simulink model "CCMHfaMotor"  (R2022b)
settings: Solver=FixedStepDiscrete  StartTime=0.0  StopTime=10.0  FixedStep=0.01  RelTol=1e-3
model workspace (MAT data in the model file):
  HfaWakeUpFlag = Simulink.Signal
  HfaWakeUpFlag.DataType = 'UInt8'
  HfaWakeUpFlag.Dimensions = 1
  HfaWakeUpFlag.DimensionsMode = 'auto'
  HfaWakeUpFlag.Complexity = 'real'
  HfaWakeUpFlag.SampleTime = -1
  HfaWakeUpFlag.SamplingMode = 'auto'
  NvMBlockHfaMtrCalData = Simulink.Signal
  NvMBlockHfaMtrCalData.DataType = 'UInt8'
  NvMBlockHfaMtrCalData.Dimensions = [200 1]
  NvMBlockHfaMtrCalData.DimensionsMode = 'auto'
  NvMBlockHfaMtrCalData.Complexity = 'real'
  NvMBlockHfaMtrCalData.SampleTime = -1
  NvMBlockHfaMtrCalData.SamplingMode = 'auto'
  NvMBlockHfaMtrCalData.InitialValue = 'NvMBlockHfaMtrCalDataInit'
  NvMBlockHfaMtrCalDataInit = Simulink.Parameter
  NvMBlockHfaMtrCalDataInit.Value = [0; 0; 0; 0; 0; 0; 0; 0; 0; 0; 0; 0; 0; 0; 0; 0; 0; 0; 0; 0; 0; 0; 0; 0; 0; 0; 0; 0; 0; 0; 0; 0; 0; 0; 0; 0; 0; 0; 0; 0; 0; 0; 0; 0; 0; 0; 0; 0; 0; 0; 0; 0; 0; 0; 0; 0; 0; 0; 0; 0; 0; 0; 0; 0; 0; 0; 0; 0; 0; 0; 0; 0; 0; 0; 0; 0; 0; 0; 0; 0; 0; 0; 0; 0; 0; 0; 0; 0; 0; 0; 0; 0; 0; 0; 0; 0; 0; 0; 0; 0; 0; 0; 0; 0; 0; 0; 0; 0; 0; 0; 0; 0; 0; 0; 0; 0; 0; 0; 0; 0; 0; 0; 0; 0; 0; 0; 0; 0; 0; 0; 0; 0; 0; 0; 0; 0; 0; 0; 0; 0; 0; 0; 0; 0; 0; 0; 0; 0; 0; 0; 0; 0; 0; 0; 0; 0; 0; 0; 0; 0; 0; 0; 0; 0; 0; 0; 0; 0; 0; 0; 0; 0; 0; 0; 0; 0; 0; 0; 0; 0; 0; 0; 0; 0; 0; 0; 0; 0; 0; 0; 0; 0; 0; 0; 0; 0; 0; 0; 0; 0]
  NvMBlockHfaMtrCalDataInit.DataType = 'UInt8'
  NvMBlockHfaMtrCalDataInit.Complexity = 'real'
  NvMBlockHfaMtrCalDataInit.Dimensions = [200 1]
undefined symbols (not defined in the file or in its folder): ComChlType, DioChannelType, HFAChlType, LinFrameType, NetworkChlType, NvMBlockId, P_DioLevelType, P_LinIfSchHandleType, P_Std_ReturnType, PowerChlType
(part 1 of 4)
settings Model: Creator=blue.ge  LastModifiedBy=blue.ge
settings DataIOCC: SaveFormat=StructureWithTime
settings OptimizationCC: DefaultParameterBehavior=Inlined  EnhancedBackFolding=on  PassReuseOutputArgsAs=Individual arguments  ZeroExternalMemoryAtStartup=off  ZeroInternalMemoryAtStartup=off  OptimizeBlockOrder=speed  DifferentSizesBufferReuse=on  OptimizationCustomize=off  EfficientTunableParamExpr=on
settings DebuggingCC: ParameterTunabilityLossMsg=error
settings ModelReferenceCC: ModelReferenceNumInstancesAllowed=Single
settings SFSimCC: MATLABDynamicMemAlloc=off
settings RTWCC: Description=AUTOSAR  SystemTargetFile=autosar.tlc  GenCodeOnly=on  TemplateMakefile=ert_default_tmf
settings CodeAppCC: ForceParamTrailComments=on  IgnoreCustomStorageClasses=off  OperatorAnnotations=on  SimulinkDataObjDesc=on  SFDataObjDesc=on  InsertBlockDesc=on
settings STFCustomTargetCC: TargetLangStandard=C99 (ISO)  MultiwordLength=256  ParMdlRefBuildCompliant=on  CompOptLevelCompliant=on  IncludeMdlTerminateFcn=off  CombineOutputUpdateFcns=on  SuppressErrorStatus=on  ERTCustomFileBanners=on  SupportNonFinite=off  SupportComplex=off  SupportContinuousTime=off  SupportNonInlinedSFcns=off  RemoveResetFunc=on  MATLABClassNameForMDSCustomization=Simulink.SoftwareTarget.ERTCustomization  AutosarCompliant=on  UseToolchainInfoCompliant=on  LookupTableObjectStructAxisOrder=2,1,3,4,...  LUTObjectStructOrderEvenSpacing=Size,Table,Breakpoints  SystemTargetFile=autosar.tlc
settings ERTTargetCC: TargetLangStandard=C99 (ISO)  ConcurrentExecutionCompliant=off  IncludeMdlTerminateFcn=off  SuppressErrorStatus=on  SupportNonFinite=off  SupportComplex=off  ModelStepFunctionPrototypeControlCompliant=off  CPPClassGenCompliant=off  AutosarCompliant=on  LookupTableObjectStructAxisOrder=2,1,3,4,...  LUTObjectStructOrderEvenSpacing=Size,Table,Breakpoints  InlinedParameterPlacement=NonHierarchical  ERTCustomFileTemplate=example_file_process.tlc

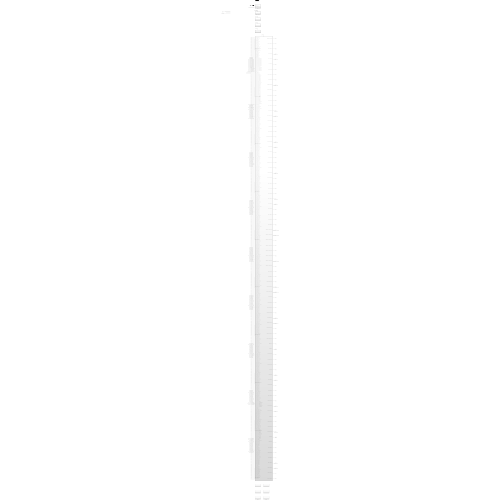
[thumbnail: Simulink's stored preview of the top-level diagram]
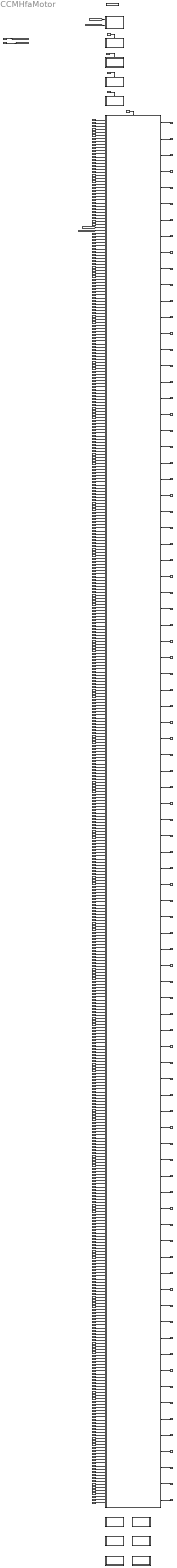
== system CCMHfaMotor ==
  CCMHfaMotor_HfaMotMode : Inport  OutputFunctionCall=on
  CCMHfaMotor_ReHvacMotMode : Inport  Port=2  OutputFunctionCall=on
  CCMHfaMotor_WtrMotMode : Inport  Port=3  OutputFunctionCall=on
  HfaMotor_50msRunnable : Inport  Port=4  SampleTime=0.05  OutputFunctionCall=on
  HfaMotor_Runnable : Inport  Port=5  SampleTime=0.02  OutputFunctionCall=on
  SbcPwrOn_SbcPwrOn : Inport  Port=6  OutDataTypeStr=PwrOnff  PortDimensions=1
  SbcWakeupSta_SbcWakeupSta : Inport  Port=7  OutDataTypeStr=Enum: SbcWakeupSta  PortDimensions=1
  AcRfrPrgSafety_AcRfrPrgSafety : Inport  Port=8  OutDataTypeStr=Enum: ActCfrPrgSafty  PortDimensions=1
  ActRfrIdeGroup_ActRfrIdeGroup : Inport  Port=9  OutDataTypeStr=Enum: ActCfrGroup  PortDimensions=1
  ActRfrIdeItem_ActRfrIdeItem : Inport  Port=10  OutDataTypeStr=Uint5  PortDimensions=1
  ActRfrIdePN_ActRfrIdePN : Inport  Port=11  OutDataTypeStr=UInt8  PortDimensions=1
  ActRfrIdeSupplier_ActRfrIdeSupplier : Inport  Port=12  OutDataTypeStr=UInt8  PortDimensions=1
  ActRfrIdeVers_ActRfrIdeVers : Inport  Port=13  OutDataTypeStr=UInt8  PortDimensions=1
  ActRfrPrgDiret_ActRfrPrgDiret : Inport  Port=14  OutDataTypeStr=Enum: ActCfrPrgDir  PortDimensions=1
  ActRfrPrgGroup_ActRfrPrgGroup : Inport  Port=15  OutDataTypeStr=Enum: ActCfrGroup  PortDimensions=1
  ActRfrPrgItem_ActRfrPrgItem : Inport  Port=16  OutDataTypeStr=Uint5  PortDimensions=1
  ActRfrPrgPrgGroup_ActRfrPrgPrgGroup : Inport  Port=17  OutDataTypeStr=Enum: ActCfrGroup  PortDimensions=1
  ActRfrPrgPrgItem_ActRfrPrgPrgItem : Inport  Port=18  OutDataTypeStr=Uint5  PortDimensions=1
  ActRfrPrgRef_ActRfrPrgRef : Inport  Port=19  OutDataTypeStr=UInt8  PortDimensions=1
  ActRfrPrgSafetyEnable_ActRfrPrgSafetyEnable : Inport  Port=20  OutDataTypeStr=Enum: ActvInActv  PortDimensions=1
  ActRfrStaCPos_ActRfrStaCPos : Inport  Port=21  OutDataTypeStr=UInt15  PortDimensions=1
  ActRfrStaEmgyRun_ActRfrStaEmgyRun : Inport  Port=22  OutDataTypeStr=Enum: ActvInActv  PortDimensions=1
  ActRfrStaErr_ActRfrStaErr : Inport  Port=23  OutDataTypeStr=Enum: ActRfrErr  PortDimensions=1
  ActRfrStaGroup_ActRfrStaGroup : Inport  Port=24  OutDataTypeStr=Enum: ActCfrGroup  PortDimensions=1
  ActRfrStaItem_ActRfrStaItem : Inport  Port=25  OutDataTypeStr=Uint5  PortDimensions=1
  ActRfrStaMod_ActRfrStaMod : Inport  Port=26  OutDataTypeStr=Enum: ActRfrMod  PortDimensions=1
  ActRfrStaReset_ActRfrStaReset : Inport  Port=27  OutDataTypeStr=Enum: ReqSts1  PortDimensions=1
  ActRfrStaStd_ActRfrStaStd : Inport  Port=28  OutDataTypeStr=Enum: YesNo2  PortDimensions=1
  BatSysBtrMSts_BatSysBtrMSts : Inport  Port=29  OutDataTypeStr=Enum: BatBtrMStsEnum  PortDimensions=1
  HfaCcmSlaveRespB0Lin4_HfaCcmSlaveRespB0Lin4 : Inport  Port=30  OutDataTypeStr=UInt8  PortDimensions=1
  HfaCcmSlaveRespB1Lin4_HfaCcmSlaveRespB1Lin4 : Inport  Port=31  OutDataTypeStr=UInt8  PortDimensions=1
  HfaCcmSlaveRespB2Lin4_HfaCcmSlaveRespB2Lin4 : Inport  Port=32  OutDataTypeStr=UInt8  PortDimensions=1
  HfaCcmSlaveRespB3Lin4_HfaCcmSlaveRespB3Lin4 : Inport  Port=33  OutDataTypeStr=UInt8  PortDimensions=1
  HfaCcmSlaveRespB4Lin4_HfaCcmSlaveRespB4Lin4 : Inport  Port=34  OutDataTypeStr=UInt8  PortDimensions=1
  HfaCcmSlaveRespB5Lin4_HfaCcmSlaveRespB5Lin4 : Inport  Port=35  OutDataTypeStr=UInt8  PortDimensions=1
  HfaCcmSlaveRespB6Lin4_HfaCcmSlaveRespB6Lin4 : Inport  Port=36  OutDataTypeStr=UInt8  PortDimensions=1
  HfaCcmSlaveRespB7Lin4_HfaCcmSlaveRespB7Lin4 : Inport  Port=37  OutDataTypeStr=UInt8  PortDimensions=1
  HmiElecAirSwngReq_HmiElecAirSwngReq : Inport  Port=38  OutDataTypeStr=Bus: HmiElecAirSwngReq  PortDimensions=1  BusOutputAsStruct=on
  HvacCfgForClimaCtrl_HvacCfgForHvacFrontBlowerType : Inport  Port=39  OutDataTypeStr=HvacCfgForHvacFrontBlowerType  PortDimensions=1
  HvacCfgForClimaCtrl_HvacCfgForHvacMiscExistSts : Inport  Port=40  OutDataTypeStr=HvacCfgForHvacMiscExistSts  PortDimensions=1
  HvacCfgForClimaCtrl_HvacCfgForHvacMotorExistSts : Inport  Port=41  OutDataTypeStr=HvacCfgForHvacMotorExistSts  PortDimensions=1
  HvacCfgForClimaCtrl_HvacCfgForHvacSensorExistSts : Inport  Port=42  OutDataTypeStr=HvacCfgForHvacSensorExistSts  PortDimensions=1
  SysServCarCfgSts_SysServCarID : Inport  Port=43  OutDataTypeStr=Enum: SysServCarID  PortDimensions=1
  SysServCarCfgSts_SysServCCPAQSSts : Inport  Port=44  OutDataTypeStr=UInt8  PortDimensions=1
  SysServCarCfgSts_SysServCCPHEXSensorSts : Inport  Port=45  OutDataTypeStr=UInt8  PortDimensions=1
  SysServCarCfgSts_SysServCCPLin2AFUSts : Inport  Port=46  OutDataTypeStr=Boolean  PortDimensions=1
  SysServCarCfgSts_SysServCCPLin2AIUSts : Inport  Port=47  OutDataTypeStr=UInt8  PortDimensions=1
  SysServCarCfgSts_SysServCCPLin2CDSSts : Inport  Port=48  OutDataTypeStr=Boolean  PortDimensions=1
  SysServCarCfgSts_SysServCCPLin2PMSISts : Inport  Port=49  OutDataTypeStr=Boolean  PortDimensions=1
  SysServCarCfgSts_SysServCCPLin3VGASts : Inport  Port=50  OutDataTypeStr=Boolean  PortDimensions=1
  SysServCarCfgSts_SysServDUAirVentSnrSts : Inport  Port=51  OutDataTypeStr=Boolean  PortDimensions=1
  SysServCarCfgSts_SysServDUBlowerPwmSignSts : Inport  Port=52  OutDataTypeStr=Boolean  PortDimensions=1
  SysServCarCfgSts_SysServDUBlowerPwmSts : Inport  Port=53  OutDataTypeStr=Boolean  PortDimensions=1
  SysServCarCfgSts_SysServDUDCDefrostSts : Inport  Port=54  OutDataTypeStr=UInt8  PortDimensions=1
  SysServCarCfgSts_SysServDUDCModeSts : Inport  Port=55  OutDataTypeStr=UInt8  PortDimensions=1
  SysServCarCfgSts_SysServDUDCRecSts : Inport  Port=56  OutDataTypeStr=UInt8  PortDimensions=1
  SysServCarCfgSts_SysServDUDCSModeSts : Inport  Port=57  OutDataTypeStr=UInt8  PortDimensions=1
  SysServCarCfgSts_SysServDUDCSTLSts : Inport  Port=58  OutDataTypeStr=UInt8  PortDimensions=1
  SysServCarCfgSts_SysServDUDCTLSts : Inport  Port=59  OutDataTypeStr=UInt8  PortDimensions=1
  SysServCarCfgSts_SysServDUDCTRSts : Inport  Port=60  OutDataTypeStr=UInt8  PortDimensions=1
  SysServCarCfgSts_SysServDUFLFootSts : Inport  Port=61  OutDataTypeStr=UInt8  PortDimensions=1
  SysServCarCfgSts_SysServDUFLVentSts : Inport  Port=62  OutDataTypeStr=UInt8  PortDimensions=1
  SysServCarCfgSts_SysServDUFRFootSts : Inport  Port=63  OutDataTypeStr=UInt8  PortDimensions=1
  SysServCarCfgSts_SysServDUFRVentSts : Inport  Port=64  OutDataTypeStr=UInt8  PortDimensions=1
  SysServCarCfgSts_SysServDUIncarMotorSts : Inport  Port=65  OutDataTypeStr=Boolean  PortDimensions=1
  SysServCarCfgSts_SysServDULin1HBMFSts : Inport  Port=66  OutDataTypeStr=Boolean  PortDimensions=1
  SysServCarCfgSts_SysServDULin4HFAFSts : Inport  Port=67  OutDataTypeStr=UInt32  PortDimensions=1
  SysServCarCfgSts_SysServDUSFLFootSts : Inport  Port=68  OutDataTypeStr=UInt8  PortDimensions=1
  SysServCarCfgSts_SysServDUSFLVentSts : Inport  Port=69  OutDataTypeStr=UInt8  PortDimensions=1
  SysServCarCfgSts_SysServDUSFRFootSts : Inport  Port=70  OutDataTypeStr=UInt8  PortDimensions=1
  SysServCarCfgSts_SysServDUSFRVentSts : Inport  Port=71  OutDataTypeStr=UInt8  PortDimensions=1
  SysServCarCfgSts_SysServDUSnsrForFCmptmtTSts : Inport  Port=72  OutDataTypeStr=UInt8  PortDimensions=1
  SysServCarCfgSts_SysServDUSnsrForRair : Inport  Port=73  OutDataTypeStr=UInt8  PortDimensions=1
  SysServCarCfgSts_SysServDUSnsrForRCmptmtTSts : Inport  Port=74  OutDataTypeStr=UInt8  PortDimensions=1
  BatLoadBtrMSts_BatLoadBtrMSts : Inport  Port=75  OutDataTypeStr=Enum: BatLoadBtrMStsEnum  PortDimensions=1
  HfaMotSetPowerOnInit_PowerOnInit : Inport  Port=76  OutDataTypeStr=Boolean  PortDimensions=1
  VehModMngtGlbSafe1_VehModMngtGlbSafe1 : Inport  Port=77  OutDataTypeStr=Bus: VehModMngtGlbSafe1  PortDimensions=1  BusOutputAsStruct=on
  HfaActr01ActPson_HfaActr01ActPson : Inport  Port=78  OutDataTypeStr=UInt16  PortDimensions=1
  HfaActr01ActPson_HfaActr01ActPson_IsUpdated : Inport  Port=79  OutDataTypeStr=boolean  PortDimensions=1
  HfaActr01BlckInd_HfaActr01BlckInd : Inport  Port=80  OutDataTypeStr=Enum: VentnActr01BlckInd  PortDimensions=1
  HfaActr01BlckSts_HfaActr01BlckSts : Inport  Port=81  OutDataTypeStr=Enum: VentnActr01BlckSts  PortDimensions=1
  HfaActr01BusAdr_HfaActr01BusAdr : Inport  Port=82  OutDataTypeStr=UInt8  PortDimensions=1
  HfaActr01CoilSts_HfaActr01CoilSts : Inport  Port=83  OutDataTypeStr=Enum: VentnMotUrgcUnlck  PortDimensions=1
  HfaActr01DrvDir_HfaActr01DrvDir : Inport  Port=84  OutDataTypeStr=Enum: VentnActr01DrvDir  PortDimensions=1
  HfaActr01ElecErr_HfaActr01ElecErr : Inport  Port=85  OutDataTypeStr=Enum: VentnActr01ElecErr  PortDimensions=1
  HfaActr01Mode_HfaActr01Mode : Inport  Port=86  OutDataTypeStr=Enum: VentnMotMode  PortDimensions=1
  HfaActr01MovDir_HfaActr01MovDir : Inport  Port=87  OutDataTypeStr=Enum: VentnMotDir  PortDimensions=1
  HfaActr01OvrTempErr_HfaActr01OvrTempErr : Inport  Port=88  OutDataTypeStr=Enum: VentnActr01OvrTempErr  PortDimensions=1
  HfaActr01PsonSts_HfaActr01PsonSts : Inport  Port=89  OutDataTypeStr=Enum: VentnMotPsonAvl  PortDimensions=1
  HfaActr01ResetSts_HfaActr01ResetSts : Inport  Port=90  OutDataTypeStr=Enum: VentnActr01ResetSts  PortDimensions=1
  HfaActr01SpclFct_HfaActr01SpclFct : Inport  Port=91  OutDataTypeStr=Enum: VentnActr01SpclFct  PortDimensions=1
  HfaActr01SpdSts_HfaActr01SpdSts : Inport  Port=92  OutDataTypeStr=Enum: VentnActr01SpdSts  PortDimensions=1
  HfaActr01TrqSts_HfaActr01TrqSts : Inport  Port=93  OutDataTypeStr=Enum: VentnMotUrgcUnlck  PortDimensions=1
  HfaActr01UrgcExist_HfaActr01UrgcExist : Inport  Port=94  OutDataTypeStr=Enum: VentnActr01UrgcExist  PortDimensions=1
  HfaActr01UrgcPson_HfaActr01UrgcPson : Inport  Port=95  OutDataTypeStr=Enum: VentnMotUrgcPson  PortDimensions=1
  HfaActr01UrgcSts_HfaActr01UrgcSts : Inport  Port=96  OutDataTypeStr=Enum: VentnMotUrgcUnlck  PortDimensions=1
  HfaActr01VoltErr_HfaActr01VoltErr : Inport  Port=97  OutDataTypeStr=Enum: VentnActr01VoltErr  PortDimensions=1
  HfaActr02ActPson_HfaActr02ActPson : Inport  Port=98  OutDataTypeStr=UInt16  PortDimensions=1
  HfaActr02ActPson_HfaActr02ActPson_IsUpdated : Inport  Port=99  OutDataTypeStr=boolean  PortDimensions=1
  HfaActr02BlckInd_HfaActr02BlckInd : Inport  Port=100  OutDataTypeStr=Enum: VentnActr01BlckInd  PortDimensions=1
  HfaActr02BlckSts_HfaActr02BlckSts : Inport  Port=101  OutDataTypeStr=Enum: VentnActr01BlckSts  PortDimensions=1
  HfaActr02BusAdr_HfaActr02BusAdr : Inport  Port=102  OutDataTypeStr=UInt8  PortDimensions=1
  HfaActr02CoilSts_HfaActr02CoilSts : Inport  Port=103  OutDataTypeStr=Enum: VentnMotUrgcUnlck  PortDimensions=1
  HfaActr02DrvDir_HfaActr02DrvDir : Inport  Port=104  OutDataTypeStr=Enum: VentnActr01DrvDir  PortDimensions=1
  HfaActr02ElecErr_HfaActr02ElecErr : Inport  Port=105  OutDataTypeStr=Enum: VentnActr01ElecErr  PortDimensions=1
  HfaActr02Mode_HfaActr02Mode : Inport  Port=106  OutDataTypeStr=Enum: VentnMotMode  PortDimensions=1
  HfaActr02MovDir_HfaActr02MovDir : Inport  Port=107  OutDataTypeStr=Enum: VentnMotDir  PortDimensions=1
  HfaActr02OvrTempErr_HfaActr02OvrTempErr : Inport  Port=108  OutDataTypeStr=Enum: VentnActr01OvrTempErr  PortDimensions=1
  HfaActr02PsonSts_HfaActr02PsonSts : Inport  Port=109  OutDataTypeStr=Enum: VentnMotPsonAvl  PortDimensions=1
  HfaActr02ResetSts_HfaActr02ResetSts : Inport  Port=110  OutDataTypeStr=Enum: VentnActr01ResetSts  PortDimensions=1
  HfaActr02SpclFct_HfaActr02SpclFct : Inport  Port=111  OutDataTypeStr=Enum: VentnActr01SpclFct  PortDimensions=1
  HfaActr02SpdSts_HfaActr02SpdSts : Inport  Port=112  OutDataTypeStr=Enum: VentnActr01SpdSts  PortDimensions=1
  HfaActr02TrqSts_HfaActr02TrqSts : Inport  Port=113  OutDataTypeStr=Enum: VentnMotUrgcUnlck  PortDimensions=1
  HfaActr02UrgcExist_HfaActr02UrgcExist : Inport  Port=114  OutDataTypeStr=Enum: VentnActr01UrgcExist  PortDimensions=1
  HfaActr02UrgcPson_HfaActr02UrgcPson : Inport  Port=115  OutDataTypeStr=Enum: VentnMotUrgcPson  PortDimensions=1
  HfaActr02UrgcSts_HfaActr02UrgcSts : Inport  Port=116  OutDataTypeStr=Enum: VentnMotUrgcUnlck  PortDimensions=1
  HfaActr02VoltErr_HfaActr02VoltErr : Inport  Port=117  OutDataTypeStr=Enum: VentnActr01VoltErr  PortDimensions=1
  HfaActr03ActPson_HfaActr03ActPson : Inport  Port=118  OutDataTypeStr=UInt16  PortDimensions=1
  HfaActr03ActPson_HfaActr03ActPson_IsUpdated : Inport  Port=119  OutDataTypeStr=boolean  PortDimensions=1
  HfaActr03BlckInd_HfaActr03BlckInd : Inport  Port=120  OutDataTypeStr=Enum: VentnActr01BlckInd  PortDimensions=1
  HfaActr03BlckSts_HfaActr03BlckSts : Inport  Port=121  OutDataTypeStr=Enum: VentnActr01BlckSts  PortDimensions=1
  HfaActr03BusAdr_HfaActr03BusAdr : Inport  Port=122  OutDataTypeStr=UInt8  PortDimensions=1
  HfaActr03CoilSts_HfaActr03CoilSts : Inport  Port=123  OutDataTypeStr=Enum: VentnMotUrgcUnlck  PortDimensions=1
  HfaActr03DrvDir_HfaActr03DrvDir : Inport  Port=124  OutDataTypeStr=Enum: VentnActr01DrvDir  PortDimensions=1
  HfaActr03ElecErr_HfaActr03ElecErr : Inport  Port=125  OutDataTypeStr=Enum: VentnActr01ElecErr  PortDimensions=1
  HfaActr03Mode_HfaActr03Mode : Inport  Port=126  OutDataTypeStr=Enum: VentnMotMode  PortDimensions=1
  HfaActr03MovDir_HfaActr03MovDir : Inport  Port=127  OutDataTypeStr=Enum: VentnMotDir  PortDimensions=1
  HfaActr03OvrTempErr_HfaActr03OvrTempErr : Inport  Port=128  OutDataTypeStr=Enum: VentnActr01OvrTempErr  PortDimensions=1
  HfaActr03PsonSts_HfaActr03PsonSts : Inport  Port=129  OutDataTypeStr=Enum: VentnMotPsonAvl  PortDimensions=1
  HfaActr03ResetSts_HfaActr03ResetSts : Inport  Port=130  OutDataTypeStr=Enum: VentnActr01ResetSts  PortDimensions=1
  HfaActr03SpclFct_HfaActr03SpclFct : Inport  Port=131  OutDataTypeStr=Enum: VentnActr01SpclFct  PortDimensions=1
  HfaActr03SpdSts_HfaActr03SpdSts : Inport  Port=132  OutDataTypeStr=Enum: VentnActr01SpdSts  PortDimensions=1
  HfaActr03TrqSts_HfaActr03TrqSts : Inport  Port=133  OutDataTypeStr=Enum: VentnMotUrgcUnlck  PortDimensions=1
  HfaActr03UrgcExist_HfaActr03UrgcExist : Inport  Port=134  OutDataTypeStr=Enum: VentnActr01UrgcExist  PortDimensions=1
  HfaActr03UrgcPson_HfaActr03UrgcPson : Inport  Port=135  OutDataTypeStr=Enum: VentnMotUrgcPson  PortDimensions=1
  HfaActr03UrgcSts_HfaActr03UrgcSts : Inport  Port=136  OutDataTypeStr=Enum: VentnMotUrgcUnlck  PortDimensions=1
  HfaActr03VoltErr_HfaActr03VoltErr : Inport  Port=137  OutDataTypeStr=Enum: VentnActr01VoltErr  PortDimensions=1
  HfaActr04ActPson_HfaActr04ActPson : Inport  Port=138  OutDataTypeStr=UInt16  PortDimensions=1
  HfaActr04ActPson_HfaActr04ActPson_IsUpdated : Inport  Port=139  OutDataTypeStr=boolean  PortDimensions=1
  HfaActr04BlckInd_HfaActr04BlckInd : Inport  Port=140  OutDataTypeStr=Enum: VentnActr01BlckInd  PortDimensions=1
  HfaActr04BlckSts_HfaActr04BlckSts : Inport  Port=141  OutDataTypeStr=Enum: VentnActr01BlckSts  PortDimensions=1
  HfaActr04BusAdr_HfaActr04BusAdr : Inport  Port=142  OutDataTypeStr=UInt8  PortDimensions=1
  HfaActr04CoilSts_HfaActr04CoilSts : Inport  Port=143  OutDataTypeStr=Enum: VentnMotUrgcUnlck  PortDimensions=1
  HfaActr04DrvDir_HfaActr04DrvDir : Inport  Port=144  OutDataTypeStr=Enum: VentnActr01DrvDir  PortDimensions=1
  HfaActr04ElecErr_HfaActr04ElecErr : Inport  Port=145  OutDataTypeStr=Enum: VentnActr01ElecErr  PortDimensions=1
  HfaActr04Mode_HfaActr04Mode : Inport  Port=146  OutDataTypeStr=Enum: VentnMotMode  PortDimensions=1
  HfaActr04MovDir_HfaActr04MovDir : Inport  Port=147  OutDataTypeStr=Enum: VentnMotDir  PortDimensions=1
  HfaActr04OvrTempErr_HfaActr04OvrTempErr : Inport  Port=148  OutDataTypeStr=Enum: VentnActr01OvrTempErr  PortDimensions=1
  HfaActr04PsonSts_HfaActr04PsonSts : Inport  Port=149  OutDataTypeStr=Enum: VentnMotPsonAvl  PortDimensions=1
  HfaActr04ResetSts_HfaActr04ResetSts : Inport  Port=150  OutDataTypeStr=Enum: VentnActr01ResetSts  PortDimensions=1
  HfaActr04SpclFct_HfaActr04SpclFct : Inport  Port=151  OutDataTypeStr=Enum: VentnActr01SpclFct  PortDimensions=1
  HfaActr04SpdSts_HfaActr04SpdSts : Inport  Port=152  OutDataTypeStr=Enum: VentnActr01SpdSts  PortDimensions=1
  HfaActr04TrqSts_HfaActr04TrqSts : Inport  Port=153  OutDataTypeStr=Enum: VentnMotUrgcUnlck  PortDimensions=1
  HfaActr04UrgcExist_HfaActr04UrgcExist : Inport  Port=154  OutDataTypeStr=Enum: VentnActr01UrgcExist  PortDimensions=1
  HfaActr04UrgcPson_HfaActr04UrgcPson : Inport  Port=155  OutDataTypeStr=Enum: VentnMotUrgcPson  PortDimensions=1
  HfaActr04UrgcSts_HfaActr04UrgcSts : Inport  Port=156  OutDataTypeStr=Enum: VentnMotUrgcUnlck  PortDimensions=1
  HfaActr04VoltErr_HfaActr04VoltErr : Inport  Port=157  OutDataTypeStr=Enum: VentnActr01VoltErr  PortDimensions=1
  HfaActr05ActPson_HfaActr05ActPson : Inport  Port=158  OutDataTypeStr=UInt16  PortDimensions=1
  HfaActr05ActPson_HfaActr05ActPson_IsUpdated : Inport  Port=159  OutDataTypeStr=boolean  PortDimensions=1
  HfaActr05BlckInd_HfaActr05BlckInd : Inport  Port=160  OutDataTypeStr=Enum: VentnActr01BlckInd  PortDimensions=1
  HfaActr05BlckSts_HfaActr05BlckSts : Inport  Port=161  OutDataTypeStr=Enum: VentnActr01BlckSts  PortDimensions=1
  HfaActr05BusAdr_HfaActr05BusAdr : Inport  Port=162  OutDataTypeStr=UInt8  PortDimensions=1
  HfaActr05CoilSts_HfaActr05CoilSts : Inport  Port=163  OutDataTypeStr=Enum: VentnMotUrgcUnlck  PortDimensions=1
  HfaActr05DrvDir_HfaActr05DrvDir : Inport  Port=164  OutDataTypeStr=Enum: VentnActr01DrvDir  PortDimensions=1
  HfaActr05ElecErr_HfaActr05ElecErr : Inport  Port=165  OutDataTypeStr=Enum: VentnActr01ElecErr  PortDimensions=1
  HfaActr05Mode_HfaActr05Mode : Inport  Port=166  OutDataTypeStr=Enum: VentnMotMode  PortDimensions=1
  HfaActr05MovDir_HfaActr05MovDir : Inport  Port=167  OutDataTypeStr=Enum: VentnMotDir  PortDimensions=1
  HfaActr05OvrTempErr_HfaActr05OvrTempErr : Inport  Port=168  OutDataTypeStr=Enum: VentnActr01OvrTempErr  PortDimensions=1
  HfaActr05PsonSts_HfaActr05PsonSts : Inport  Port=169  OutDataTypeStr=Enum: VentnMotPsonAvl  PortDimensions=1
  HfaActr05ResetSts_HfaActr05ResetSts : Inport  Port=170  OutDataTypeStr=Enum: VentnActr01ResetSts  PortDimensions=1
  HfaActr05SpclFct_HfaActr05SpclFct : Inport  Port=171  OutDataTypeStr=Enum: VentnActr01SpclFct  PortDimensions=1
  HfaActr05SpdSts_HfaActr05SpdSts : Inport  Port=172  OutDataTypeStr=Enum: VentnActr01SpdSts  PortDimensions=1
  HfaActr05TrqSts_HfaActr05TrqSts : Inport  Port=173  OutDataTypeStr=Enum: VentnMotUrgcUnlck  PortDimensions=1
  HfaActr05UrgcExist_HfaActr05UrgcExist : Inport  Port=174  OutDataTypeStr=Enum: VentnActr01UrgcExist  PortDimensions=1
  HfaActr05UrgcPson_HfaActr05UrgcPson : Inport  Port=175  OutDataTypeStr=Enum: VentnMotUrgcPson  PortDimensions=1
  HfaActr05UrgcSts_HfaActr05UrgcSts : Inport  Port=176  OutDataTypeStr=Enum: VentnMotUrgcUnlck  PortDimensions=1
  HfaActr05VoltErr_HfaActr05VoltErr : Inport  Port=177  OutDataTypeStr=Enum: VentnActr01VoltErr  PortDimensions=1
  HfaActr06ActPson_HfaActr06ActPson : Inport  Port=178  OutDataTypeStr=UInt16  PortDimensions=1
  HfaActr06ActPson_HfaActr06ActPson_IsUpdated : Inport  Port=179  OutDataTypeStr=boolean  PortDimensions=1
  HfaActr06BlckInd_HfaActr06BlckInd : Inport  Port=180  OutDataTypeStr=Enum: VentnActr01BlckInd  PortDimensions=1
  HfaActr06BlckSts_HfaActr06BlckSts : Inport  Port=181  OutDataTypeStr=Enum: VentnActr01BlckSts  PortDimensions=1
  HfaActr06BusAdr_HfaActr06BusAdr : Inport  Port=182  OutDataTypeStr=UInt8  PortDimensions=1
  HfaActr06CoilSts_HfaActr06CoilSts : Inport  Port=183  OutDataTypeStr=Enum: VentnMotUrgcUnlck  PortDimensions=1
  HfaActr06DrvDir_HfaActr06DrvDir : Inport  Port=184  OutDataTypeStr=Enum: VentnActr01DrvDir  PortDimensions=1
  HfaActr06ElecErr_HfaActr06ElecErr : Inport  Port=185  OutDataTypeStr=Enum: VentnActr01ElecErr  PortDimensions=1
  HfaActr06Mode_HfaActr06Mode : Inport  Port=186  OutDataTypeStr=Enum: VentnMotMode  PortDimensions=1
  HfaActr06MovDir_HfaActr06MovDir : Inport  Port=187  OutDataTypeStr=Enum: VentnMotDir  PortDimensions=1
  HfaActr06OvrTempErr_HfaActr06OvrTempErr : Inport  Port=188  OutDataTypeStr=Enum: VentnActr01OvrTempErr  PortDimensions=1
  HfaActr06PsonSts_HfaActr06PsonSts : Inport  Port=189  OutDataTypeStr=Enum: VentnMotPsonAvl  PortDimensions=1
  HfaActr06ResetSts_HfaActr06ResetSts : Inport  Port=190  OutDataTypeStr=Enum: VentnActr01ResetSts  PortDimensions=1
  HfaActr06SpclFct_HfaActr06SpclFct : Inport  Port=191  OutDataTypeStr=Enum: VentnActr01SpclFct  PortDimensions=1
  HfaActr06SpdSts_HfaActr06SpdSts : Inport  Port=192  OutDataTypeStr=Enum: VentnActr01SpdSts  PortDimensions=1
  HfaActr06TrqSts_HfaActr06TrqSts : Inport  Port=193  OutDataTypeStr=Enum: VentnMotUrgcUnlck  PortDimensions=1
  HfaActr06UrgcExist_HfaActr06UrgcExist : Inport  Port=194  OutDataTypeStr=Enum: VentnActr01UrgcExist  PortDimensions=1
  HfaActr06UrgcPson_HfaActr06UrgcPson : Inport  Port=195  OutDataTypeStr=Enum: VentnMotUrgcPson  PortDimensions=1
  HfaActr06UrgcSts_HfaActr06UrgcSts : Inport  Port=196  OutDataTypeStr=Enum: VentnMotUrgcUnlck  PortDimensions=1
  HfaActr06VoltErr_HfaActr06VoltErr : Inport  Port=197  OutDataTypeStr=Enum: VentnActr01VoltErr  PortDimensions=1
  HfaActr07ActPson_HfaActr07ActPson : Inport  Port=198  OutDataTypeStr=UInt16  PortDimensions=1
  HfaActr07ActPson_HfaActr07ActPson_IsUpdated : Inport  Port=199  OutDataTypeStr=boolean  PortDimensions=1
  HfaActr07BlckInd_HfaActr07BlckInd : Inport  Port=200  OutDataTypeStr=Enum: VentnActr01BlckInd  PortDimensions=1
  HfaActr07BlckSts_HfaActr07BlckSts : Inport  Port=201  OutDataTypeStr=Enum: VentnActr01BlckSts  PortDimensions=1
  HfaActr07BusAdr_HfaActr07BusAdr : Inport  Port=202  OutDataTypeStr=UInt8  PortDimensions=1
  HfaActr07CoilSts_HfaActr07CoilSts : Inport  Port=203  OutDataTypeStr=Enum: VentnMotUrgcUnlck  PortDimensions=1
  HfaActr07DrvDir_HfaActr07DrvDir : Inport  Port=204  OutDataTypeStr=Enum: VentnActr01DrvDir  PortDimensions=1
  HfaActr07ElecErr_HfaActr07ElecErr : Inport  Port=205  OutDataTypeStr=Enum: VentnActr01ElecErr  PortDimensions=1
  HfaActr07Mode_HfaActr07Mode : Inport  Port=206  OutDataTypeStr=Enum: VentnMotMode  PortDimensions=1
  HfaActr07MovDir_HfaActr07MovDir : Inport  Port=207  OutDataTypeStr=Enum: VentnMotDir  PortDimensions=1
  HfaActr07OvrTempErr_HfaActr07OvrTempErr : Inport  Port=208  OutDataTypeStr=Enum: VentnActr01OvrTempErr  PortDimensions=1
  HfaActr07PsonSts_HfaActr07PsonSts : Inport  Port=209  OutDataTypeStr=Enum: VentnMotPsonAvl  PortDimensions=1
  HfaActr07ResetSts_HfaActr07ResetSts : Inport  Port=210  OutDataTypeStr=Enum: VentnActr01ResetSts  PortDimensions=1
  HfaActr07SpclFct_HfaActr07SpclFct : Inport  Port=211  OutDataTypeStr=Enum: VentnActr01SpclFct  PortDimensions=1
  HfaActr07SpdSts_HfaActr07SpdSts : Inport  Port=212  OutDataTypeStr=Enum: VentnActr01SpdSts  PortDimensions=1
  HfaActr07TrqSts_HfaActr07TrqSts : Inport  Port=213  OutDataTypeStr=Enum: VentnMotUrgcUnlck  PortDimensions=1
  HfaActr07UrgcExist_HfaActr07UrgcExist : Inport  Port=214  OutDataTypeStr=Enum: VentnActr01UrgcExist  PortDimensions=1
  HfaActr07UrgcPson_HfaActr07UrgcPson : Inport  Port=215  OutDataTypeStr=Enum: VentnMotUrgcPson  PortDimensions=1
  HfaActr07UrgcSts_HfaActr07UrgcSts : Inport  Port=216  OutDataTypeStr=Enum: VentnMotUrgcUnlck  PortDimensions=1
  HfaActr07VoltErr_HfaActr07VoltErr : Inport  Port=217  OutDataTypeStr=Enum: VentnActr01VoltErr  PortDimensions=1
  HfaActr08ActPson_HfaActr08ActPson : Inport  Port=218  OutDataTypeStr=UInt16  PortDimensions=1
  HfaActr08ActPson_HfaActr08ActPson_IsUpdated : Inport  Port=219  OutDataTypeStr=boolean  PortDimensions=1
  HfaActr08BlckInd_HfaActr08BlckInd : Inport  Port=220  OutDataTypeStr=Enum: VentnActr01BlckInd  PortDimensions=1
  HfaActr08BlckSts_HfaActr08BlckSts : Inport  Port=221  OutDataTypeStr=Enum: VentnActr01BlckSts  PortDimensions=1
  HfaActr08BusAdr_HfaActr08BusAdr : Inport  Port=222  OutDataTypeStr=UInt8  PortDimensions=1
  HfaActr08CoilSts_HfaActr08CoilSts : Inport  Port=223  OutDataTypeStr=Enum: VentnMotUrgcUnlck  PortDimensions=1
  HfaActr08DrvDir_HfaActr08DrvDir : Inport  Port=224  OutDataTypeStr=Enum: VentnActr01DrvDir  PortDimensions=1
  HfaActr08ElecErr_HfaActr08ElecErr : Inport  Port=225  OutDataTypeStr=Enum: VentnActr01ElecErr  PortDimensions=1
  HfaActr08Mode_HfaActr08Mode : Inport  Port=226  OutDataTypeStr=Enum: VentnMotMode  PortDimensions=1
  HfaActr08MovDir_HfaActr08MovDir : Inport  Port=227  OutDataTypeStr=Enum: VentnMotDir  PortDimensions=1
  HfaActr08OvrTempErr_HfaActr08OvrTempErr : Inport  Port=228  OutDataTypeStr=Enum: VentnActr01OvrTempErr  PortDimensions=1
  HfaActr08PsonSts_HfaActr08PsonSts : Inport  Port=229  OutDataTypeStr=Enum: VentnMotPsonAvl  PortDimensions=1
  HfaActr08ResetSts_HfaActr08ResetSts : Inport  Port=230  OutDataTypeStr=Enum: VentnActr01ResetSts  PortDimensions=1
  HfaActr08SpclFct_HfaActr08SpclFct : Inport  Port=231  OutDataTypeStr=Enum: VentnActr01SpclFct  PortDimensions=1
  HfaActr08SpdSts_HfaActr08SpdSts : Inport  Port=232  OutDataTypeStr=Enum: VentnActr01SpdSts  PortDimensions=1
  HfaActr08TrqSts_HfaActr08TrqSts : Inport  Port=233  OutDataTypeStr=Enum: VentnMotUrgcUnlck  PortDimensions=1
  HfaActr08UrgcExist_HfaActr08UrgcExist : Inport  Port=234  OutDataTypeStr=Enum: VentnActr01UrgcExist  PortDimensions=1
  HfaActr08UrgcPson_HfaActr08UrgcPson : Inport  Port=235  OutDataTypeStr=Enum: VentnMotUrgcPson  PortDimensions=1
  HfaActr08UrgcSts_HfaActr08UrgcSts : Inport  Port=236  OutDataTypeStr=Enum: VentnMotUrgcUnlck  PortDimensions=1
  HfaActr08VoltErr_HfaActr08VoltErr : Inport  Port=237  OutDataTypeStr=Enum: VentnActr01VoltErr  PortDimensions=1
  HfaActr09ActPson_HfaActr09ActPson : Inport  Port=238  OutDataTypeStr=UInt16  PortDimensions=1
  HfaActr09ActPson_HfaActr09ActPson_IsUpdated : Inport  Port=239  OutDataTypeStr=boolean  PortDimensions=1
  HfaActr09BlckInd_HfaActr09BlckInd : Inport  Port=240  OutDataTypeStr=Enum: VentnActr01BlckInd  PortDimensions=1
  HfaActr09BlckSts_HfaActr09BlckSts : Inport  Port=241  OutDataTypeStr=Enum: VentnActr01BlckSts  PortDimensions=1
  HfaActr09BusAdr_HfaActr09BusAdr : Inport  Port=242  OutDataTypeStr=UInt8  PortDimensions=1
  HfaActr09CoilSts_HfaActr09CoilSts : Inport  Port=243  OutDataTypeStr=Enum: VentnMotUrgcUnlck  PortDimensions=1
  HfaActr09DrvDir_HfaActr09DrvDir : Inport  Port=244  OutDataTypeStr=Enum: VentnActr01DrvDir  PortDimensions=1
  HfaActr09ElecErr_HfaActr09ElecErr : Inport  Port=245  OutDataTypeStr=Enum: VentnActr01ElecErr  PortDimensions=1
  HfaActr09Mode_HfaActr09Mode : Inport  Port=246  OutDataTypeStr=Enum: VentnMotMode  PortDimensions=1
  HfaActr09MovDir_HfaActr09MovDir : Inport  Port=247  OutDataTypeStr=Enum: VentnMotDir  PortDimensions=1
  HfaActr09OvrTempErr_HfaActr09OvrTempErr : Inport  Port=248  OutDataTypeStr=Enum: VentnActr01OvrTempErr  PortDimensions=1
  HfaActr09PsonSts_HfaActr09PsonSts : Inport  Port=249  OutDataTypeStr=Enum: VentnMotPsonAvl  PortDimensions=1
  HfaActr09ResetSts_HfaActr09ResetSts : Inport  Port=250  OutDataTypeStr=Enum: VentnActr01ResetSts  PortDimensions=1
  HfaActr09SpclFct_HfaActr09SpclFct : Inport  Port=251  OutDataTypeStr=Enum: VentnActr01SpclFct  PortDimensions=1
  HfaActr09SpdSts_HfaActr09SpdSts : Inport  Port=252  OutDataTypeStr=Enum: VentnActr01SpdSts  PortDimensions=1
  HfaActr09TrqSts_HfaActr09TrqSts : Inport  Port=253  OutDataTypeStr=Enum: VentnMotUrgcUnlck  PortDimensions=1
  HfaActr09UrgcExist_HfaActr09UrgcExist : Inport  Port=254  OutDataTypeStr=Enum: VentnActr01UrgcExist  PortDimensions=1
  HfaActr09UrgcPson_HfaActr09UrgcPson : Inport  Port=255  OutDataTypeStr=Enum: VentnMotUrgcPson  PortDimensions=1
  HfaActr09UrgcSts_HfaActr09UrgcSts : Inport  Port=256  OutDataTypeStr=Enum: VentnMotUrgcUnlck  PortDimensions=1
  HfaActr09VoltErr_HfaActr09VoltErr : Inport  Port=257  OutDataTypeStr=Enum: VentnActr01VoltErr  PortDimensions=1
  HfaActr10ActPson_HfaActr10ActPson : Inport  Port=258  OutDataTypeStr=UInt16  PortDimensions=1
  HfaActr10ActPson_HfaActr10ActPson_IsUpdated : Inport  Port=259  OutDataTypeStr=boolean  PortDimensions=1
  HfaActr10BlckInd_HfaActr10BlckInd : Inport  Port=260  OutDataTypeStr=Enum: VentnActr01BlckInd  PortDimensions=1
  HfaActr10BlckSts_HfaActr10BlckSts : Inport  Port=261  OutDataTypeStr=Enum: VentnActr01BlckSts  PortDimensions=1
  HfaActr10BusAdr_HfaActr10BusAdr : Inport  Port=262  OutDataTypeStr=UInt8  PortDimensions=1
  HfaActr10CoilSts_HfaActr10CoilSts : Inport  Port=263  OutDataTypeStr=Enum: VentnMotUrgcUnlck  PortDimensions=1
  HfaActr10DrvDir_HfaActr10DrvDir : Inport  Port=264  OutDataTypeStr=Enum: VentnActr01DrvDir  PortDimensions=1
  HfaActr10ElecErr_HfaActr10ElecErr : Inport  Port=265  OutDataTypeStr=Enum: VentnActr01ElecErr  PortDimensions=1
  HfaActr10Mode_HfaActr10Mode : Inport  Port=266  OutDataTypeStr=Enum: VentnMotMode  PortDimensions=1
  HfaActr10MovDir_HfaActr10MovDir : Inport  Port=267  OutDataTypeStr=Enum: VentnMotDir  PortDimensions=1
  HfaActr10OvrTempErr_HfaActr10OvrTempErr : Inport  Port=268  OutDataTypeStr=Enum: VentnActr01OvrTempErr  PortDimensions=1
  HfaActr10PsonSts_HfaActr10PsonSts : Inport  Port=269  OutDataTypeStr=Enum: VentnMotPsonAvl  PortDimensions=1
  HfaActr10ResetSts_HfaActr10ResetSts : Inport  Port=270  OutDataTypeStr=Enum: VentnActr01ResetSts  PortDimensions=1
  HfaActr10SpclFct_HfaActr10SpclFct : Inport  Port=271  OutDataTypeStr=Enum: VentnActr01SpclFct  PortDimensions=1
  HfaActr10SpdSts_HfaActr10SpdSts : Inport  Port=272  OutDataTypeStr=Enum: VentnActr01SpdSts  PortDimensions=1
  HfaActr10TrqSts_HfaActr10TrqSts : Inport  Port=273  OutDataTypeStr=Enum: VentnMotUrgcUnlck  PortDimensions=1
  HfaActr10UrgcExist_HfaActr10UrgcExist : Inport  Port=274  OutDataTypeStr=Enum: VentnActr01UrgcExist  PortDimensions=1
  HfaActr10UrgcPson_HfaActr10UrgcPson : Inport  Port=275  OutDataTypeStr=Enum: VentnMotUrgcPson  PortDimensions=1
  HfaActr10UrgcSts_HfaActr10UrgcSts : Inport  Port=276  OutDataTypeStr=Enum: VentnMotUrgcUnlck  PortDimensions=1
  HfaActr10VoltErr_HfaActr10VoltErr : Inport  Port=277  OutDataTypeStr=Enum: VentnActr01VoltErr  PortDimensions=1
  HfaActr11ActPson_HfaActr11ActPson : Inport  Port=278  OutDataTypeStr=UInt16  PortDimensions=1
  HfaActr11ActPson_HfaActr11ActPson_IsUpdated : Inport  Port=279  OutDataTypeStr=boolean  PortDimensions=1
  HfaActr11BlckInd_HfaActr11BlckInd : Inport  Port=280  OutDataTypeStr=Enum: VentnActr01BlckInd  PortDimensions=1
  HfaActr11BlckSts_HfaActr11BlckSts : Inport  Port=281  OutDataTypeStr=Enum: VentnActr01BlckSts  PortDimensions=1
  HfaActr11BusAdr_HfaActr11BusAdr : Inport  Port=282  OutDataTypeStr=UInt8  PortDimensions=1
  HfaActr11CoilSts_HfaActr11CoilSts : Inport  Port=283  OutDataTypeStr=Enum: VentnMotUrgcUnlck  PortDimensions=1
  HfaActr11DrvDir_HfaActr11DrvDir : Inport  Port=284  OutDataTypeStr=Enum: VentnActr01DrvDir  PortDimensions=1
  HfaActr11ElecErr_HfaActr11ElecErr : Inport  Port=285  OutDataTypeStr=Enum: VentnActr01ElecErr  PortDimensions=1
  HfaActr11Mode_HfaActr11Mode : Inport  Port=286  OutDataTypeStr=Enum: VentnMotMode  PortDimensions=1
  HfaActr11MovDir_HfaActr11MovDir : Inport  Port=287  OutDataTypeStr=Enum: VentnMotDir  PortDimensions=1
  HfaActr11OvrTempErr_HfaActr11OvrTempErr : Inport  Port=288  OutDataTypeStr=Enum: VentnActr01OvrTempErr  PortDimensions=1
  HfaActr11PsonSts_HfaActr11PsonSts : Inport  Port=289  OutDataTypeStr=Enum: VentnMotPsonAvl  PortDimensions=1
  HfaActr11ResetSts_HfaActr11ResetSts : Inport  Port=290  OutDataTypeStr=Enum: VentnActr01ResetSts  PortDimensions=1
  HfaActr11SpclFct_HfaActr11SpclFct : Inport  Port=291  OutDataTypeStr=Enum: VentnActr01SpclFct  PortDimensions=1
  HfaActr11SpdSts_HfaActr11SpdSts : Inport  Port=292  OutDataTypeStr=Enum: VentnActr01SpdSts  PortDimensions=1
  HfaActr11TrqSts_HfaActr11TrqSts : Inport  Port=293  OutDataTypeStr=Enum: VentnMotUrgcUnlck  PortDimensions=1
  HfaActr11UrgcExist_HfaActr11UrgcExist : Inport  Port=294  OutDataTypeStr=Enum: VentnActr01UrgcExist  PortDimensions=1
  HfaActr11UrgcPson_HfaActr11UrgcPson : Inport  Port=295  OutDataTypeStr=Enum: VentnMotUrgcPson  PortDimensions=1
  HfaActr11UrgcSts_HfaActr11UrgcSts : Inport  Port=296  OutDataTypeStr=Enum: VentnMotUrgcUnlck  PortDimensions=1
  HfaActr11VoltErr_HfaActr11VoltErr : Inport  Port=297  OutDataTypeStr=Enum: VentnActr01VoltErr  PortDimensions=1
  HfaActr12ActPson_HfaActr12ActPson : Inport  Port=298  OutDataTypeStr=UInt16  PortDimensions=1
  HfaActr12ActPson_HfaActr12ActPson_IsUpdated : Inport  Port=299  OutDataTypeStr=boolean  PortDimensions=1
  HfaActr12BlckInd_HfaActr12BlckInd : Inport  Port=300  OutDataTypeStr=Enum: VentnActr01BlckInd  PortDimensions=1
  HfaActr12BlckSts_HfaActr12BlckSts : Inport  Port=301  OutDataTypeStr=Enum: VentnActr01BlckSts  PortDimensions=1
  HfaActr12BusAdr_HfaActr12BusAdr : Inport  Port=302  OutDataTypeStr=UInt8  PortDimensions=1
  HfaActr12CoilSts_HfaActr12CoilSts : Inport  Port=303  OutDataTypeStr=Enum: VentnMotUrgcUnlck  PortDimensions=1
  HfaActr12DrvDir_HfaActr12DrvDir : Inport  Port=304  OutDataTypeStr=Enum: VentnActr01DrvDir  PortDimensions=1
  HfaActr12ElecErr_HfaActr12ElecErr : Inport  Port=305  OutDataTypeStr=Enum: VentnActr01ElecErr  PortDimensions=1
  HfaActr12Mode_HfaActr12Mode : Inport  Port=306  OutDataTypeStr=Enum: VentnMotMode  PortDimensions=1
  HfaActr12MovDir_HfaActr12MovDir : Inport  Port=307  OutDataTypeStr=Enum: VentnMotDir  PortDimensions=1
  HfaActr12OvrTempErr_HfaActr12OvrTempErr : Inport  Port=308  OutDataTypeStr=Enum: VentnActr01OvrTempErr  PortDimensions=1
  HfaActr12PsonSts_HfaActr12PsonSts : Inport  Port=309  OutDataTypeStr=Enum: VentnMotPsonAvl  PortDimensions=1
  HfaActr12ResetSts_HfaActr12ResetSts : Inport  Port=310  OutDataTypeStr=Enum: VentnActr01ResetSts  PortDimensions=1
  HfaActr12SpclFct_HfaActr12SpclFct : Inport  Port=311  OutDataTypeStr=Enum: VentnActr01SpclFct  PortDimensions=1
  HfaActr12SpdSts_HfaActr12SpdSts : Inport  Port=312  OutDataTypeStr=Enum: VentnActr01SpdSts  PortDimensions=1
  HfaActr12TrqSts_HfaActr12TrqSts : Inport  Port=313  OutDataTypeStr=Enum: VentnMotUrgcUnlck  PortDimensions=1
  HfaActr12UrgcExist_HfaActr12UrgcExist : Inport  Port=314  OutDataTypeStr=Enum: VentnActr01UrgcExist  PortDimensions=1
  HfaActr12UrgcPson_HfaActr12UrgcPson : Inport  Port=315  OutDataTypeStr=Enum: VentnMotUrgcPson  PortDimensions=1
  HfaActr12UrgcSts_HfaActr12UrgcSts : Inport  Port=316  OutDataTypeStr=Enum: VentnMotUrgcUnlck  PortDimensions=1
  HfaActr12VoltErr_HfaActr12VoltErr : Inport  Port=317  OutDataTypeStr=Enum: VentnActr01VoltErr  PortDimensions=1
  HfaActr13ActPson_HfaActr13ActPson : Inport  Port=318  OutDataTypeStr=UInt16  PortDimensions=1
  HfaActr13ActPson_HfaActr13ActPson_IsUpdated : Inport  Port=319  OutDataTypeStr=boolean  PortDimensions=1
  HfaActr13BlckInd_HfaActr13BlckInd : Inport  Port=320  OutDataTypeStr=Enum: VentnActr01BlckInd  PortDimensions=1
  HfaActr13BlckSts_HfaActr13BlckSts : Inport  Port=321  OutDataTypeStr=Enum: VentnActr01BlckSts  PortDimensions=1
  HfaActr13BusAdr_HfaActr13BusAdr : Inport  Port=322  OutDataTypeStr=UInt8  PortDimensions=1
  HfaActr13CoilSts_HfaActr13CoilSts : Inport  Port=323  OutDataTypeStr=Enum: VentnMotUrgcUnlck  PortDimensions=1
  HfaActr13DrvDir_HfaActr13DrvDir : Inport  Port=324  OutDataTypeStr=Enum: VentnActr01DrvDir  PortDimensions=1
  HfaActr13ElecErr_HfaActr13ElecErr : Inport  Port=325  OutDataTypeStr=Enum: VentnActr01ElecErr  PortDimensions=1
  HfaActr13Mode_HfaActr13Mode : Inport  Port=326  OutDataTypeStr=Enum: VentnMotMode  PortDimensions=1
  HfaActr13MovDir_HfaActr13MovDir : Inport  Port=327  OutDataTypeStr=Enum: VentnMotDir  PortDimensions=1
  HfaActr13OvrTempErr_HfaActr13OvrTempErr : Inport  Port=328  OutDataTypeStr=Enum: VentnActr01OvrTempErr  PortDimensions=1
  HfaActr13PsonSts_HfaActr13PsonSts : Inport  Port=329  OutDataTypeStr=Enum: VentnMotPsonAvl  PortDimensions=1
  HfaActr13ResetSts_HfaActr13ResetSts : Inport  Port=330  OutDataTypeStr=Enum: VentnActr01ResetSts  PortDimensions=1
  HfaActr13SpclFct_HfaActr13SpclFct : Inport  Port=331  OutDataTypeStr=Enum: VentnActr01SpclFct  PortDimensions=1
  HfaActr13SpdSts_HfaActr13SpdSts : Inport  Port=332  OutDataTypeStr=Enum: VentnActr01SpdSts  PortDimensions=1
  HfaActr13TrqSts_HfaActr13TrqSts : Inport  Port=333  OutDataTypeStr=Enum: VentnMotUrgcUnlck  PortDimensions=1
  HfaActr13UrgcExist_HfaActr13UrgcExist : Inport  Port=334  OutDataTypeStr=Enum: VentnActr01UrgcExist  PortDimensions=1
  HfaActr13UrgcPson_HfaActr13UrgcPson : Inport  Port=335  OutDataTypeStr=Enum: VentnMotUrgcPson  PortDimensions=1
  HfaActr13UrgcSts_HfaActr13UrgcSts : Inport  Port=336  OutDataTypeStr=Enum: VentnMotUrgcUnlck  PortDimensions=1
  HfaActr13VoltErr_HfaActr13VoltErr : Inport  Port=337  OutDataTypeStr=Enum: VentnActr01VoltErr  PortDimensions=1
  HfaActr14ActPson_HfaActr14ActPson : Inport  Port=338  OutDataTypeStr=UInt16  PortDimensions=1
  HfaActr14ActPson_HfaActr14ActPson_IsUpdated : Inport  Port=339  OutDataTypeStr=boolean  PortDimensions=1
  HfaActr14BlckInd_HfaActr14BlckInd : Inport  Port=340  OutDataTypeStr=Enum: VentnActr01BlckInd  PortDimensions=1